simulink model "TosAeb"  (R2021a)
settings: Solver=FixedStepDiscrete  StartTime=0.0  StopTime=inf  FixedStep=0.01  RelTol=1e-3
undefined symbols (not defined in the file or in its folder): ACCModSts, AccSts1, AEB_ObjectMovingOrientation, AEB_ObjectMovingState, CN_BTCActive, CN_CurrentGearR, CN_CurrentGearR_DHT, CN_PTCActive, CN_VDCActive, CN_VSC_EspOff, CN_VSC_FCW_AEB_Error, CN_VSC_FCW_AEB_NoDispay, CN_VSC_FCW_AEB_PerfmDgdtn, CN_VSC_FCW_AEB_TempUnAvlbl, CN_VSC_VehStandstill, CNaccelAvoidMax, CNaccelAvoidMin, CNbrakePedalPress, CNcomfortStrLeftAccelMax, CNcomfortStrLeftAccelMin, CNcomfortStrRightAccelMax, CNcomfortStrRightAccelMin, CNDrvDoorOpen, CNDrvModeEco, CNDrvModeNorm, CNDrvModeOther, CNDrvModeSport, CNDrvSBRreminder, CNNull, CNobjInvalidID, CNPassDoorOpen, CNPassSBRreminder, CNPEBSAccelNegInf, CNPEBSAccelNull, CNPEBSTimeInf, CNPEBSTimeNull, CNSTOPMAX, CNSTOPNULL, CNsubjWidth, CNSwtOFF, CNSwtON, CNTTCMAX, CNTTCNULL, CNVehConfigAEBCycNone, CNVehConfigAEBNone, CNVehConfigAEBPedNone, CNVehConfigFCWNone, CNVehConfigJANone, CollisionSeverity, FALSE, FCWSnvty, JACycObjCalcInfoDefaultParams, JAPedObjCalcInfoDefaultParams, JAVehObjCalcInfoDefaultParams, latCycObjCalcInfoDefaultParams, latPedObjCalcInfoDefaultParams, longObjCalcInfoDefaultParams, ModSts, movingStatus, ObjectType ... (+1112)
libraries: simulink x3031, AEBSFunctionLib x50, Ta_Library x33, AEBSStateMachineLib x23, Mylibrary x12, simulink_extras x7, ObjSelect_lib x3, dspstat3 x2
(part 11 of 102)

== system TosAeb/FcwAebJa_Step_sys/ALG_AEBSystem/ALG_AEBOutput/AEBS_DVRInfoCalc/AEB_DVR_INFOMap ==
  AEB_LON_DVR_INFO : Inport
  AEB_LATPED_DVR_INFO : Inport  Port=2
  AEB_LATCYC_DVR_INFO : Inport  Port=3
  AEB_JAVEH_DVR_INFO : Inport  Port=4
  AEB_JAPED_DVR_INFO : Inport  Port=5
  Bus Creator : BusCreator  Inputs=62  OutDataTypeStr=Bus: AEB_DVR_INFO_BUS
      settings: InheritFromInputs=off
  Bus Selector : BusSelector  OutputSignals=AEBObjID,AEBObjVideoCfmd,AEBObjState,AEBObjDx,AEBObjDy,AEBObjVx,AEBObjVy,AEBObjAx,AEBObjAy,AEBObjExist,AEBObjObstcl,AEBObjCntAlive,AEBObjOverlap,AEBObjDrvby
  Bus Selector1 : BusSelector  OutputSignals=PedObjID,PedObjVideoCfmd,PedObjState,PedObjDx,PedObjDy,PedObjVx,PedObjVy,PedObjAx,PedObjAy,PedObjExist,PedObjObstcl,PedObjCntAlive
  Bus Selector2 : BusSelector  OutputSignals=CyclistObjID,CyclistObjVideoCfmd,CyclistObjState,CyclistObjDx,CyclistObjDy,CyclistObjVx,CyclistObjVy,CyclistObjAx,CyclistObjAy,CyclistObjExist,CyclistObjObstcl,CyclistObjCntAlive
  Bus Selector3 : BusSelector  OutputSignals=AEBJAObjID,AEBJAObjVideoCfmd,AEBJAObjState,AEBJAObjDx,AEBJAObjDy,AEBJAObjVx,AEBJAObjVy,AEBJAObjAx,AEBJAObjAy,AEBJAObjExist,AEBJAObjObstcl,AEBJAObjCntAlive
  Bus Selector4 : BusSelector  OutputSignals=JAPedObjID,JAPedObjVideoCfmd,JAPedObjState,JAPedObjDx,JAPedObjDy,JAPedObjVx,JAPedObjVy,JAPedObjAx,JAPedObjAy,JAPedObjExist,JAPedObjObstcl,JAPedObjCntAlive
  Data Type Conversion{1..2,6,8..14,16,20..31,34..45,48..56,59..63,65} (50 alike) : DataTypeConversion
  Data Type Conversion{7,15,19,32..33,46..47,57..58,64} (10 alike) : DataTypeConversion  OutDataTypeStr=uint16
  Data Type Conversion17 : DataTypeConversion  OutDataTypeStr=uint8
  Data Type Conversion18 : DataTypeConversion  OutDataTypeStr=uint8
  AEB_DVR_INFO : Outport  OutDataTypeStr=Bus: AEB_DVR_INFO_BUS
  wires:
  Bus Selector4/12 -> Data Type Conversion64/1  "<JAPedObjCntAlive>"
  Bus Selector4/11 -> Data Type Conversion65/1  "<JAPedObjObstcl>"
  Bus Selector4/10 -> Data Type Conversion60/1  "<JAPedObjExist>"
  Bus Selector4/9 -> Data Type Conversion63/1  "<JAPedObjAy>"
  Bus Selector4/8 -> Data Type Conversion62/1  "<JAPedObjAx>"
  Bus Selector4/7 -> Data Type Conversion61/1  "<JAPedObjVy>"
  Bus Selector4/6 -> Data Type Conversion52/1  "<JAPedObjVx>"
  Bus Selector4/5 -> Data Type Conversion55/1  "<JAPedObjDy>"
  Bus Selector4/4 -> Data Type Conversion54/1  "<JAPedObjDx>"
  Bus Selector4/3 -> Data Type Conversion53/1  "<JAPedObjState>"
  Bus Selector4/2 -> Data Type Conversion51/1  "<JAPedObjVideoCfmd>"
  Bus Selector4/1 -> Data Type Conversion58/1  "<JAPedObjID>"
  AEB_JAPED_DVR_INFO/1 -> Bus Selector4/1
  Bus Selector3/12 -> Data Type Conversion57/1  "<AEBJAObjCntAlive>"
  Bus Selector3/11 -> Data Type Conversion56/1  "<AEBJAObjObstcl>"
  Bus Selector3/10 -> Data Type Conversion59/1  "<AEBJAObjExist>"
  Bus Selector3/9 -> Data Type Conversion50/1  "<AEBJAObjAy>"
  Bus Selector3/8 -> Data Type Conversion49/1  "<AEBJAObjAx>"
  Bus Selector3/7 -> Data Type Conversion48/1  "<AEBJAObjVy>"
  Bus Selector3/6 -> Data Type Conversion41/1  "<AEBJAObjVx>"
  Bus Selector3/5 -> Data Type Conversion44/1  "<AEBJAObjDy>"
  Bus Selector3/4 -> Data Type Conversion43/1  "<AEBJAObjDx>"
  Bus Selector3/3 -> Data Type Conversion42/1  "<AEBJAObjState>"
  Bus Selector3/2 -> Data Type Conversion40/1  "<AEBJAObjVideoCfmd>"
  Bus Selector3/1 -> Data Type Conversion47/1  "<AEBJAObjID>"
  AEB_JAVEH_DVR_INFO/1 -> Bus Selector3/1
  Bus Selector2/12 -> Data Type Conversion46/1  "<CyclistObjCntAlive>"
  Bus Selector2/11 -> Data Type Conversion45/1  "<CyclistObjObstcl>"
  Bus Selector2/10 -> Data Type Conversion6/1  "<CyclistObjExist>"
  Bus Selector2/9 -> Data Type Conversion39/1  "<CyclistObjAy>"
  Bus Selector2/8 -> Data Type Conversion38/1  "<CyclistObjAx>"
  Bus Selector2/7 -> Data Type Conversion37/1  "<CyclistObjVy>"
  Bus Selector2/6 -> Data Type Conversion27/1  "<CyclistObjVx>"
  Bus Selector2/5 -> Data Type Conversion30/1  "<CyclistObjDy>"
  Bus Selector2/4 -> Data Type Conversion29/1  "<CyclistObjDx>"
  Bus Selector2/3 -> Data Type Conversion28/1  "<CyclistObjState>"
  Bus Selector2/2 -> Data Type Conversion26/1  "<CyclistObjVideoCfmd>"
  Bus Selector2/1 -> Data Type Conversion33/1  "<CyclistObjID>"
  AEB_LATCYC_DVR_INFO/1 -> Bus Selector2/1
  Bus Selector1/12 -> Data Type Conversion32/1  "<PedObjCntAlive>"
  Bus Selector1/11 -> Data Type Conversion31/1  "<PedObjObstcl>"
  Bus Selector1/10 -> Data Type Conversion2/1  "<PedObjExist>"
  Bus Selector1/9 -> Data Type Conversion25/1  "<PedObjAy>"
  Bus Selector1/8 -> Data Type Conversion24/1  "<PedObjAx>"
  Bus Selector1/7 -> Data Type Conversion23/1  "<PedObjVy>"
  Bus Selector1/6 -> Data Type Conversion1/1  "<PedObjVx>"
  Bus Selector1/1 -> Data Type Conversion19/1  "<PedObjID>"
  Bus Selector1/5 -> Data Type Conversion36/1  "<PedObjDy>"
  Bus Selector1/2 -> Data Type Conversion16/1  "<PedObjVideoCfmd>"
  Bus Selector1/4 -> Data Type Conversion35/1  "<PedObjDx>"
  Bus Selector1/3 -> Data Type Conversion34/1  "<PedObjState>"
  AEB_LATPED_DVR_INFO/1 -> Bus Selector1/1
  Bus Selector/14 -> Data Type Conversion18/1  "<AEBObjDrvby>"
  Bus Selector/13 -> Data Type Conversion17/1  "<AEBObjOverlap>"
  Bus Selector/12 -> Data Type Conversion15/1  "<AEBObjCntAlive>"
  Bus Selector/11 -> Data Type Conversion22/1  "<AEBObjObstcl>"
  Bus Selector/10 -> Data Type Conversion21/1  "<AEBObjExist>"
  Bus Selector/9 -> Data Type Conversion20/1  "<AEBObjAy>"
  Bus Selector/8 -> Data Type Conversion11/1  "<AEBObjAx>"
  Bus Selector/7 -> Data Type Conversion14/1  "<AEBObjVy>"
  Bus Selector/6 -> Data Type Conversion13/1  "<AEBObjVx>"
  Bus Selector/5 -> Data Type Conversion12/1  "<AEBObjDy>"
  Bus Selector/4 -> Data Type Conversion10/1  "<AEBObjDx>"
  Bus Selector/3 -> Data Type Conversion9/1  "<AEBObjState>"
  Bus Selector/2 -> Data Type Conversion8/1  "<AEBObjVideoCfmd>"
  Bus Selector/1 -> Data Type Conversion7/1  "<AEBObjID>"
  AEB_LON_DVR_INFO/1 -> Bus Selector/1
  Data Type Conversion24/1 -> Bus Creator/22  "PedObjAx"
  Data Type Conversion16/1 -> Bus Creator/16  "PedObjVideoCfmd"
  Data Type Conversion20/1 -> Bus Creator/9  "AEBObjAy"
  Data Type Conversion47/1 -> Bus Creator/39  "AEBJAObjID"
  Data Type Conversion38/1 -> Bus Creator/34  "CyclistObjAx"
  Data Type Conversion11/1 -> Bus Creator/8  "AEBObjAx"
  Data Type Conversion17/1 -> Bus Creator/13  "AEBObjOverlap"
  Data Type Conversion55/1 -> Bus Creator/55  "JAPedObjDy"
  Data Type Conversion22/1 -> Bus Creator/11  "AEBObjObstcl"
  Data Type Conversion19/1 -> Bus Creator/15  "PedObjID"
  Data Type Conversion9/1 -> Bus Creator/3  "AEBObjState"
  Data Type Conversion53/1 -> Bus Creator/53  "JAPedObjState"
  Data Type Conversion60/1 -> Bus Creator/60  "JAPedObjExist"
  Data Type Conversion33/1 -> Bus Creator/27  "CyclistObjID"
  Data Type Conversion56/1 -> Bus Creator/49  "AEBJAObjObstcl"
  Data Type Conversion36/1 -> Bus Creator/19  "PedObjDy"
  Data Type Conversion57/1 -> Bus Creator/50  "AEBJAObjCntAlive"
  Data Type Conversion6/1 -> Bus Creator/36  "CyclistObjExist"
  Data Type Conversion10/1 -> Bus Creator/4  "AEBObjDx"
  Data Type Conversion52/1 -> Bus Creator/56  "JAPedObjVx"
  Data Type Conversion8/1 -> Bus Creator/2  "AEBObjVideoCfmd"
  Data Type Conversion15/1 -> Bus Creator/12  "AEBObjCntAlive"
  Data Type Conversion18/1 -> Bus Creator/14  "AEBObjDrvby"
  Data Type Conversion41/1 -> Bus Creator/44  "AEBJAObjVx"
  Data Type Conversion21/1 -> Bus Creator/10  "AEBObjExist"
  Bus Creator/1 -> AEB_DVR_INFO/1
  Data Type Conversion14/1 -> Bus Creator/7  "AEBObjVy"
  Data Type Conversion34/1 -> Bus Creator/17  "PedObjState"
  Data Type Conversion23/1 -> Bus Creator/21  "PedObjVy"
  Data Type Conversion42/1 -> Bus Creator/41  "AEBJAObjState"
  Data Type Conversion2/1 -> Bus Creator/24  "PedObjExist"
  Data Type Conversion35/1 -> Bus Creator/18  "PedObjDx"
  Data Type Conversion31/1 -> Bus Creator/25  "PedObjObstcl"
  Data Type Conversion28/1 -> Bus Creator/29  "CyclistObjState"
  Data Type Conversion7/1 -> Bus Creator/1  "AEBObjID"
  Data Type Conversion49/1 -> Bus Creator/46  "AEBJAObjAx"
  Data Type Conversion61/1 -> Bus Creator/57  "JAPedObjVy"
  Data Type Conversion39/1 -> Bus Creator/35  "CyclistObjAy"
  Data Type Conversion59/1 -> Bus Creator/48  "AEBJAObjExist"
  Data Type Conversion62/1 -> Bus Creator/58  "JAPedObjAx"
  Data Type Conversion43/1 -> Bus Creator/42  "AEBJAObjDx"
  Data Type Conversion46/1 -> Bus Creator/38  "CyclistObjCntAlive"
  Data Type Conversion44/1 -> Bus Creator/43  "AEBJAObjDy"
  Data Type Conversion30/1 -> Bus Creator/31  "CyclistObjDy"
  Data Type Conversion63/1 -> Bus Creator/59  "JAPedObjAy"
  Data Type Conversion50/1 -> Bus Creator/47  "AEBJAObjAy"
  Data Type Conversion27/1 -> Bus Creator/32  "CyclistObjVx"
  Data Type Conversion45/1 -> Bus Creator/37  "CyclistObjObstcl"
  Data Type Conversion26/1 -> Bus Creator/28  "CyclistObjVideoCfmd"
  Data Type Conversion65/1 -> Bus Creator/61  "JAPedObjObstcl"
  Data Type Conversion13/1 -> Bus Creator/6  "AEBObjVx"
  Data Type Conversion29/1 -> Bus Creator/30  "CyclistObjDx"
  Data Type Conversion32/1 -> Bus Creator/26  "PedObjCntAlive"
  Data Type Conversion40/1 -> Bus Creator/40  "AEBJAObjVideoCfmd"
  Data Type Conversion64/1 -> Bus Creator/62  "JAPedObjCntAlive"
  Data Type Conversion12/1 -> Bus Creator/5  "AEBObjDy"
  Data Type Conversion58/1 -> Bus Creator/51  "JAPedObjID"
  Data Type Conversion25/1 -> Bus Creator/23  "PedObjAy"
  Data Type Conversion48/1 -> Bus Creator/45  "AEBJAObjVy"
  Data Type Conversion1/1 -> Bus Creator/20  "PedObjVx"
  Data Type Conversion54/1 -> Bus Creator/54  "JAPedObjDx"
  Data Type Conversion51/1 -> Bus Creator/52  "JAPedObjVideoCfmd"
  Data Type Conversion37/1 -> Bus Creator/33  "CyclistObjVy"

== system TosAeb/FcwAebJa_Step_sys/ALG_AEBSystem/ALG_AEBOutput/AEBS_DVRInfoCalc/AEB_JAPED_DVR_INFO ==
  VehConfigAEBJA : Inport
  JAPedTgtCalcInfo : Inport  Port=2
  Compare To Constant : simulink/Logic and Bit Operations/Compare To Constant  relop===  const=FALSE  OutDataTypeStr=boolean
  If : If  IfExpression=u1
  If Action Subsystem : Subsystem  TreatAsAtomicUnit=on
  Merge : Merge
  Subsystem : Subsystem
  AEB_JAPED_DVR_INFO : Outport
  wires:
  Merge/1 -> AEB_JAPED_DVR_INFO/1
  Subsystem/1(AEB_JAPED_DVR_INFO) -> Merge/2
  If Action Subsystem/1(AEB_JAPED_DVR_INFO) -> Merge/1
  If/2 -> Subsystem/Action
  If/1 -> If Action Subsystem/Action
  Compare To Constant/1 -> If/1
  VehConfigAEBJA/1 -> Compare To Constant/1
  JAPedTgtCalcInfo/1 -> Subsystem/1(JAPedTgtCalcInfo)

== system TosAeb/FcwAebJa_Step_sys/ALG_AEBSystem/ALG_AEBOutput/AEBS_DVRInfoCalc/AEB_JAVEH_DVR_INFO ==
  JAVehTgtCalcInfo : Inport
  VehConfigAEBJA : Inport  Port=2
  Compare To Constant : simulink/Logic and Bit Operations/Compare To Constant  relop===  const=FALSE  OutDataTypeStr=boolean
  If : If  IfExpression=u1
  If Action Subsystem : Subsystem  TreatAsAtomicUnit=on
  Merge : Merge
  Subsystem : Subsystem
  AEB_JAVEH_DVR_INFO : Outport
  wires:
  Merge/1 -> AEB_JAVEH_DVR_INFO/1
  Subsystem/1(AEB_JAVEH_DVR_INFO) -> Merge/2
  If Action Subsystem/1(AEB_JAVEH_DVR_INFO) -> Merge/1
  If/2 -> Subsystem/Action
  If/1 -> If Action Subsystem/Action
  JAVehTgtCalcInfo/1 -> Subsystem/1(JAVehTgtCalcInfo)
  Compare To Constant/1 -> If/1
  VehConfigAEBJA/1 -> Compare To Constant/1

== system TosAeb/FcwAebJa_Step_sys/ALG_AEBSystem/ALG_AEBOutput/AEBS_DVRInfoCalc/AEB_LATCYC_DVR_INFO ==
  VehicleConfigAEBCyc : Inport
  latCycTgtCalcInfo : Inport  Port=2
  Compare To Constant : simulink/Logic and Bit Operations/Compare To Constant  relop===  const=FALSE  OutDataTypeStr=boolean
  If : If  IfExpression=u1
  If Action Subsystem : Subsystem  TreatAsAtomicUnit=on
  Merge : Merge
  Subsystem : Subsystem
  AEB_LATCYC_DVR_INFO : Outport
  wires:
  Merge/1 -> AEB_LATCYC_DVR_INFO/1
  Subsystem/1(AEB_LATCYC_DVR_INFO) -> Merge/2
  If Action Subsystem/1(AEB_LATCYC_DVR_INFO) -> Merge/1
  If/2 -> Subsystem/Action
  If/1 -> If Action Subsystem/Action
  Compare To Constant/1 -> If/1
  VehicleConfigAEBCyc/1 -> Compare To Constant/1
  latCycTgtCalcInfo/1 -> Subsystem/1(latCycTgtCalcInfo)

== system TosAeb/FcwAebJa_Step_sys/ALG_AEBSystem/ALG_AEBOutput/AEBS_DVRInfoCalc/AEB_LATPED_DVR_INFO ==
  VehicleConfigAEBPed : Inport
  latPedTgtCalcInfo : Inport  Port=2
  Compare To Constant : simulink/Logic and Bit Operations/Compare To Constant  relop===  const=FALSE  OutDataTypeStr=boolean
  If : If  IfExpression=u1
  If Action Subsystem : Subsystem  TreatAsAtomicUnit=on
  Merge : Merge
  Subsystem : Subsystem
  AEB_LATPED_DVR_INFO : Outport
  wires:
  Merge/1 -> AEB_LATPED_DVR_INFO/1
  Subsystem/1(AEB_LATPED_DVR_INFO) -> Merge/2
  If Action Subsystem/1(AEB_LATPED_DVR_INFO) -> Merge/1
  If/2 -> Subsystem/Action
  If/1 -> If Action Subsystem/Action
  VehicleConfigAEBPed/1 -> Compare To Constant/1
  Compare To Constant/1 -> If/1
  latPedTgtCalcInfo/1 -> Subsystem/1(latPedTgtCalcInfo)

== system TosAeb/FcwAebJa_Step_sys/ALG_AEBSystem/ALG_AEBOutput/AEBS_DVRInfoCalc/AEB_LON_DVR_INFO ==
  VehicleConfigFCW : Inport
  VehicleConfigAEB : Inport  Port=2
  longObjCalcInfo : Inport  Port=3
  Compare To Constant : simulink/Logic and Bit Operations/Compare To Constant  relop===  const=FALSE  OutDataTypeStr=boolean
  If : If  IfExpression=u1
  If Action Subsystem : Subsystem  TreatAsAtomicUnit=on
  If Action Subsystem1 : Subsystem  TreatAsAtomicUnit=on
  Logical Operator : Logic  Operator=OR  AllPortsSameDT=off  OutDataTypeStr=boolean
  Merge : Merge
  AEB_LON_DVR_INFO : Outport
  wires:
  Compare To Constant/1 -> If/1
  VehicleConfigAEB/1 -> Logical Operator/2
  Logical Operator/1 -> Compare To Constant/1
  VehicleConfigFCW/1 -> Logical Operator/1
  If Action Subsystem/1(AEB_LON_DVR_INFO) -> Merge/2
  longObjCalcInfo/1 -> If Action Subsystem/1(longObjCalcInfo)
  If Action Subsystem1/1(AEB_LON_DVR_INFO) -> Merge/1
  If/2 -> If Action Subsystem/Action
  If/1 -> If Action Subsystem1/Action
  Merge/1 -> AEB_LON_DVR_INFO/1

== system TosAeb/FcwAebJa_Step_sys/ALG_AEBSystem/ALG_AEBOutput/AEBS_IPHUTInfoCalc/AEBS_IPHUTInfoMap ==
  note: AEB_LePedDetn
      |
      | AEB_LePedTyp
      |
      | AEB_LePedHeading
      |
      | AEB_LePedDx
      |
      | AEB_LePedDy
      |
      | AEB_LePed_collisionprob
      |
      | AEB_RiPedDetn
      |
      | AEB_RiPedTyp
      |
      | AEB_RiPedHeading
      |
      | AEB_RiPedDx
      |
      | AEB_RiPedDy
      |
      | AEB_RiPed_collisionprob
      |
      | AEB_LeCyclistDetn
      |
      | AEB_LeCyclistTyp
      |
      | AEB_LeCyclistHeading
      |
      | AEB_LeCyclistDx
      |
      | AEB_LeCyclistDy
      |
      | AEB_LeCyclist_collisionprob
      |
      | AEB_RiCyclistDetn
      |
      | AEB_RiCyclistTyp
      |
      | AEB_RiCyclistHeading
      |
      | AEB_RiCyclistDx
      |
      | AEB_RiCyclistDy
      |
      | AEB_RiCyclist_collisionprob
      |
      | FCWAEB_longTTC
      |
      | FCWAEB_crossingTTC
      |
      | FCWAEB_JATTC
  note: 0x0:No Warning
      | 0x1:Warning
  note: 0x0:No Warning
      | 0x1:LattenWarning
  AEB_VehResp : Inport
  AEB_PedResp : Inport  Port=2
  FCWSnvty : Inport  Port=3
  AEB_JAResp : Inport  Port=4
  FCW_AEB_VehFctSts : Inport  Port=5
  FCW_AEB_PedFctSts : Inport  Port=6
  FCW_AEB_JAFctSts : Inport  Port=7
  AEB_VehTrig : Inport  Port=8
  AEB_PedTrig : Inport  Port=9
  AEB_JABrkTrig : Inport  Port=10
  AEB_ObjSty : Inport  Port=11
  FCW_Warn : Inport  Port=12
  Latten_Warn : Inport  Port=13
  AR_HUD_Info : Inport  Port=14
  Bus Creator : BusCreator  Inputs=40  OutDataTypeStr=Bus: AEB_IPHUT_INFO_BUS  NonVirtualBus=on
      settings: InheritFromInputs=off
  Bus Selector : BusSelector  OutputSignals=AEB_LePedDetn,AEB_LePedTyp,AEB_LePedHeading,AEB_LePedDx,AEB_LePedDy,AEB_LePed_collisionprob,AEB_RiPedDetn,AEB_RiPedTyp,AEB_RiPedHeading,AEB_RiPedDx,AEB_RiPedDy,AEB_RiPed_collisionprob,AEB_LeCyclistDetn,AEB_LeCyclistTyp,AEB_LeCyclistHeading,AEB_LeCyclistDx,AEB_LeCyclistDy,AEB_LeCyclist_collisionprob,AEB_RiCyclistDetn,AEB_RiCyclistTyp,AEB_RiCyclistHeading,AEB_RiCyclistDx,AEB_RiCyclistDy,AEB_RiCyclist_collisionprob,FCWAEB_longTTC,FCWAEB_crossingTTC,FCWAEB_JATTC
  Data Type Conversion{,1..5,34} (7 alike) : DataTypeConversion  OutDataTypeStr=uint8  SaturateOnIntegerOverflow=on
  Data Type Conversion{6..33} (28 alike) : DataTypeConversion
  Signal Conversion{,1..39} (40 alike) : SignalConversion  OverrideOpt=off
  AEB_IPHUT_INFO : Outport  OutDataTypeStr=Bus: AEB_IPHUT_INFO_BUS
  wires:
  Bus Selector/27 -> Data Type Conversion32/1  "<FCWAEB_JATTC>"
  Bus Selector/26 -> Data Type Conversion31/1  "<FCWAEB_crossingTTC>"
  Bus Selector/25 -> Data Type Conversion26/1  "<FCWAEB_longTTC>"
  Bus Selector/24 -> Data Type Conversion30/1  "<AEB_RiCyclist_collisionprob>"
  Bus Selector/23 -> Data Type Conversion29/1  "<AEB_RiCyclistDy>"
  Bus Selector/22 -> Data Type Conversion28/1  "<AEB_RiCyclistDx>"
  Bus Selector/21 -> Data Type Conversion27/1  "<AEB_RiCyclistHeading>"
  Bus Selector/20 -> Data Type Conversion21/1  "<AEB_RiCyclistTyp>"
  Bus Selector/19 -> Data Type Conversion25/1  "<AEB_RiCyclistDetn>"
  Bus Selector/18 -> Data Type Conversion24/1  "<AEB_LeCyclist_collisionprob>"
  Bus Selector/17 -> Data Type Conversion23/1  "<AEB_LeCyclistDy>"
  Bus Selector/16 -> Data Type Conversion22/1  "<AEB_LeCyclistDx>"
  Bus Selector/15 -> Data Type Conversion16/1  "<AEB_LeCyclistHeading>"
  Bus Selector/14 -> Data Type Conversion20/1  "<AEB_LeCyclistTyp>"
  Bus Selector/13 -> Data Type Conversion19/1  "<AEB_LeCyclistDetn>"
  Bus Selector/12 -> Data Type Conversion18/1  "<AEB_RiPed_collisionprob>"
  Bus Selector/11 -> Data Type Conversion17/1  "<AEB_RiPedDy>"
  Bus Selector/10 -> Data Type Conversion11/1  "<AEB_RiPedDx>"
  Bus Selector/9 -> Data Type Conversion15/1  "<AEB_RiPedHeading>"
  Bus Selector/8 -> Data Type Conversion14/1  "<AEB_RiPedTyp>"
  Bus Selector/7 -> Data Type Conversion13/1  "<AEB_RiPedDetn>"
  Bus Selector/6 -> Data Type Conversion12/1  "<AEB_LePed_collisionprob>"
  Bus Selector/5 -> Data Type Conversion10/1  "<AEB_LePedDy>"
  Bus Selector/4 -> Data Type Conversion9/1  "<AEB_LePedDx>"
  Bus Selector/3 -> Data Type Conversion8/1  "<AEB_LePedHeading>"
  Bus Selector/2 -> Data Type Conversion7/1  "<AEB_LePedTyp>"
  Bus Selector/1 -> Data Type Conversion6/1  "<AEB_LePedDetn>"
  AR_HUD_Info/1 -> Bus Selector/1
  Data Type Conversion33/1 -> Signal Conversion12/1
  Data Type Conversion32/1 -> Signal Conversion39/1
  Data Type Conversion31/1 -> Signal Conversion38/1
  Data Type Conversion30/1 -> Signal Conversion36/1
  Data Type Conversion28/1 -> Signal Conversion34/1
  Data Type Conversion29/1 -> Signal Conversion35/1
  Data Type Conversion26/1 -> Signal Conversion37/1
  Data Type Conversion27/1 -> Signal Conversion33/1
  Data Type Conversion23/1 -> Signal Conversion29/1
  Data Type Conversion22/1 -> Signal Conversion28/1
  Data Type Conversion24/1 -> Signal Conversion30/1
  Data Type Conversion21/1 -> Signal Conversion32/1
  Data Type Conversion25/1 -> Signal Conversion31/1
  Data Type Conversion18/1 -> Signal Conversion24/1
  Data Type Conversion19/1 -> Signal Conversion25/1
  Data Type Conversion17/1 -> Signal Conversion23/1
  Data Type Conversion16/1 -> Signal Conversion27/1
  Data Type Conversion20/1 -> Signal Conversion26/1
  Data Type Conversion11/1 -> Signal Conversion22/1
  Data Type Conversion12/1 -> Signal Conversion18/1
  Data Type Conversion14/1 -> Signal Conversion20/1
  Data Type Conversion13/1 -> Signal Conversion19/1
  Data Type Conversion15/1 -> Signal Conversion21/1
  Data Type Conversion10/1 -> Signal Conversion17/1
  Data Type Conversion9/1 -> Signal Conversion16/1
  Data Type Conversion8/1 -> Signal Conversion15/1
  Data Type Conversion7/1 -> Signal Conversion14/1
  Data Type Conversion6/1 -> Signal Conversion13/1
  Signal Conversion39/1 -> Bus Creator/40  "FCWAEB_JATTC"
  Signal Conversion35/1 -> Bus Creator/36  "AEB_RiCyclistDy"
  Signal Conversion36/1 -> Bus Creator/37  "AEB_RiCyclist_collisionprob"
  Signal Conversion37/1 -> Bus Creator/38  "FCWAEB_longTTC"
  Signal Conversion38/1 -> Bus Creator/39  "FCWAEB_crossingTTC"
  Signal Conversion34/1 -> Bus Creator/35  "AEB_RiCyclistDx"
  Signal Conversion33/1 -> Bus Creator/34  "AEB_RiCyclistHeading"
  Signal Conversion32/1 -> Bus Creator/33  "AEB_RiCyclistTyp"
  Signal Conversion31/1 -> Bus Creator/32  "AEB_RiCyclistDetn"
  Signal Conversion30/1 -> Bus Creator/31  "AEB_LeCyclist_collisionprob"
  Signal Conversion29/1 -> Bus Creator/30  "AEB_LeCyclistDy"
  Signal Conversion28/1 -> Bus Creator/29  "AEB_LeCyclistDx"
  Signal Conversion27/1 -> Bus Creator/28  "AEB_LeCyclistHeading"
  Signal Conversion26/1 -> Bus Creator/27  "AEB_LeCyclistTyp"
  Signal Conversion25/1 -> Bus Creator/26  "AEB_LeCyclistDetn"
  Signal Conversion24/1 -> Bus Creator/25  "AEB_RiPed_collisionprob"
  Signal Conversion23/1 -> Bus Creator/24  "AEB_RiPedDy"
  Signal Conversion22/1 -> Bus Creator/23  "AEB_RiPedDx"
  Signal Conversion21/1 -> Bus Creator/22  "AEB_RiPedHeading"
  Signal Conversion20/1 -> Bus Creator/21  "AEB_RiPedTyp"
  Signal Conversion19/1 -> Bus Creator/20  "AEB_RiPedDetn"
  Signal Conversion18/1 -> Bus Creator/19  "AEB_LePed_collisionprob"
  Signal Conversion17/1 -> Bus Creator/18  "AEB_LePedDy"
  Signal Conversion16/1 -> Bus Creator/17  "AEB_LePedDx"
  Signal Conversion15/1 -> Bus Creator/16  "AEB_LePedHeading"
  Signal Conversion14/1 -> Bus Creator/15  "AEB_LePedTyp"
  Signal Conversion13/1 -> Bus Creator/14  "AEB_LePedDetn"
  Data Type Conversion5/1 -> Signal Conversion6/1
  Data Type Conversion4/1 -> Signal Conversion10/1
  Data Type Conversion2/1 -> Signal Conversion9/1
  Data Type Conversion/1 -> Signal Conversion8/1
  Data Type Conversion3/1 -> Signal Conversion7/1
  Data Type Conversion1/1 -> Signal Conversion2/1
  Data Type Conversion34/1 -> Signal Conversion11/1
  AEB_VehTrig/1 -> Signal Conversion4/1
  FCW_Warn/1 -> Data Type Conversion2/1
  AEB_PedTrig/1 -> Signal Conversion5/1
  FCW_AEB_VehFctSts/1 -> Data Type Conversion3/1
  AEB_VehResp/1 -> Signal Conversion/1
  AEB_JAResp/1 -> Signal Conversion3/1
  AEB_PedResp/1 -> Signal Conversion1/1
  FCWSnvty/1 -> Data Type Conversion1/1
  FCW_AEB_JAFctSts/1 -> Data Type Conversion4/1
  FCW_AEB_PedFctSts/1 -> Data Type Conversion/1
  AEB_ObjSty/1 -> Data Type Conversion5/1
  Signal Conversion12/1 -> Bus Creator/10  "AEB_JABrkTrig"
  AEB_JABrkTrig/1 -> Data Type Conversion33/1
  Signal Conversion11/1 -> Bus Creator/12  "Latten_Warn"
  Signal Conversion10/1 -> Bus Creator/7  "AEB_JAFuncSts"
  Signal Conversion1/1 -> Bus Creator/2  "AEB_PedResp"
  Signal Conversion5/1 -> Bus Creator/9  "AEB_PedTrig"
  Bus Creator/1 -> AEB_IPHUT_INFO/1
  Signal Conversion9/1 -> Bus Creator/11  "FCW_Warn"
  Signal Conversion8/1 -> Bus Creator/6  "FCW_AEB_PedFctSts"
  Signal Conversion2/1 -> Bus Creator/4  "FCW_Snvty"
  Signal Conversion7/1 -> Bus Creator/5  "FCW_AEB_VehFctSts"
  Signal Conversion3/1 -> Bus Creator/3  "AEB_JA_Resp"
  Signal Conversion6/1 -> Bus Creator/13  "AEB_ObjSty"
  Signal Conversion/1 -> Bus Creator/1  "AEB_VehResp"
  Signal Conversion4/1 -> Bus Creator/8  "AEB_VehTrig"
  Latten_Warn/1 -> Data Type Conversion34/1

== system TosAeb/FcwAebJa_Step_sys/ALG_AEBSystem/ALG_AEBOutput/AEBS_IPHUTInfoCalc/AEB_ObjStyMap ==
  AEBTrigger : Inport
  AEBpartFuncImpArb : Inport  Port=2
  AEBfullFuncImpArb : Inport  Port=3
  EBAFuncImpArb : Inport  Port=4
  cycAEBpartFuncImpArb : Inport  Port=5
  cycAEBfullFuncImpArb : Inport  Port=6
  pedAEBfullFuncImpArb : Inport  Port=7
  JA_AEBfullFuncImpArb : Inport  Port=8
  JA_pedAEBfullFuncImpArb : Inport  Port=9
  longObjectType : Inport  Port=10
  latPedObjectType : Inport  Port=11
  latCycObjectType : Inport  Port=12
  JaPedObjectType : Inport  Port=13
  JaVehObjectType : Inport  Port=14
  AEB_ObjStyFlow : Stateflow chart  TreatAsAtomicUnit=on
  AEB_ObjSty : Outport
  wires:
  JaVehObjectType/1 -> AEB_ObjStyFlow/14(JaVehObjectType)
  JaPedObjectType/1 -> AEB_ObjStyFlow/13(JaPedObjectType)
  latCycObjectType/1 -> AEB_ObjStyFlow/12(latCycObjectType)
  latPedObjectType/1 -> AEB_ObjStyFlow/11(latPedObjectType)
  longObjectType/1 -> AEB_ObjStyFlow/10(longObjectType)
  AEB_ObjStyFlow/1(Inter_AEB_ObjSty) -> AEB_ObjSty/1
  cycAEBfullFuncImpArb/1 -> AEB_ObjStyFlow/6(cycAEBpartFuncImpArb)
  cycAEBpartFuncImpArb/1 -> AEB_ObjStyFlow/5(pedAEBfullFuncImpArb)
  EBAFuncImpArb/1 -> AEB_ObjStyFlow/4(AEBfullFuncImpArb)
  AEBfullFuncImpArb/1 -> AEB_ObjStyFlow/3(EBAfullFuncImpArb)
  AEBTrigger/1 -> AEB_ObjStyFlow/1(AEBTrigger)
  AEBpartFuncImpArb/1 -> AEB_ObjStyFlow/2(AEBPartFuncImpArb)
  JA_AEBfullFuncImpArb/1 -> AEB_ObjStyFlow/8(JA_AEBfullFuncImpArb)
  JA_pedAEBfullFuncImpArb/1 -> AEB_ObjStyFlow/9(JA_pedAEBfullFuncImpArb)
  pedAEBfullFuncImpArb/1 -> AEB_ObjStyFlow/7(cycAEBfullFuncImpArb)

== system TosAeb/FcwAebJa_Step_sys/ALG_AEBSystem/ALG_AEBOutput/AEBS_IPHUTInfoCalc/AEB_ObjStyMap1 ==
  AEBTrigger : Inport
  AEBpartFuncImpArb : Inport  Port=2
  AEBfullFuncImpArb : Inport  Port=3
  EBAFuncImpArb : Inport  Port=4
  cycAEBpartFuncImpArb : Inport  Port=5
  cycAEBfullFuncImpArb : Inport  Port=6
  pedAEBfullFuncImpArb : Inport  Port=7
  JA_AEBfullFuncImpArb : Inport  Port=8
  JA_pedAEBfullFuncImpArb : Inport  Port=9
  FCTBFuncImpArb : Inport  Port=10
  AEB_ObjStyFlow : Stateflow chart  TreatAsAtomicUnit=on
  AEB_ObjSty : Outport
  wires:
  FCTBFuncImpArb/1 -> AEB_ObjStyFlow/10(FCTBFuncImpArb)
  pedAEBfullFuncImpArb/1 -> AEB_ObjStyFlow/7(cycAEBfullFuncImpArb)
  JA_pedAEBfullFuncImpArb/1 -> AEB_ObjStyFlow/9(JA_pedAEBfullFuncImpArb)
  JA_AEBfullFuncImpArb/1 -> AEB_ObjStyFlow/8(JA_AEBfullFuncImpArb)
  AEBpartFuncImpArb/1 -> AEB_ObjStyFlow/2(AEBPartFuncImpArb)
  AEBTrigger/1 -> AEB_ObjStyFlow/1(AEBTrigger)
  AEBfullFuncImpArb/1 -> AEB_ObjStyFlow/3(EBAfullFuncImpArb)
  EBAFuncImpArb/1 -> AEB_ObjStyFlow/4(AEBfullFuncImpArb)
  cycAEBpartFuncImpArb/1 -> AEB_ObjStyFlow/5(pedAEBfullFuncImpArb)
  cycAEBfullFuncImpArb/1 -> AEB_ObjStyFlow/6(cycAEBpartFuncImpArb)
  AEB_ObjStyFlow/1(AEB_ObjSty) -> AEB_ObjSty/1

== system TosAeb/FcwAebJa_Step_sys/ALG_AEBSystem/ALG_AEBOutput/AEBS_IPHUTInfoCalc/AEB_TrigMap ==
  note: 0x0:Not tirggered
      | 0x1:AEB_JA Function Triggered for vehicle
      | 0x2:AEB_JA Function Triggered for pedestrian
      | 0x3:reverse
  note: AEB_VehTrigMap
  note: AEB_PedTrigMap
  note: AEB_JABrkTrigMap
  AEBTrigger : Inport
  AEBLonObjectType : Inport  Port=2
  EBAfullFuncImpArb : Inport  Port=3
  AEBfullFuncImpArb : Inport  Port=4
  AEBPartFuncImpArb : Inport  Port=5
  pedAEBfullFuncImpArb : Inport  Port=6
  cycAEBfullFuncImpArb : Inport  Port=7
  cycAEBpartFuncImpArb : Inport  Port=8
  JA_pedAEBfullFuncImpArb : Inport  Port=9
  JA_AEBfullFuncImpArb : Inport  Port=10
  Compare To Constant{,1..2,4..6,9..10} (8 alike) : simulink/Logic and Bit Operations/Compare To Constant  relop===  const=ModSts.ActiveState  OutDataTypeStr=boolean
  Compare To Constant{7..8,11,14} (4 alike) : simulink/Logic and Bit Operations/Compare To Constant  relop===  const=TRUE  OutDataTypeStr=boolean
  Compare To Constant12 : simulink/Logic and Bit Operations/Compare To Constant  relop===  const=ObjectType.BICYCLE  OutDataTypeStr=boolean
  Compare To Constant13 : simulink/Logic and Bit Operations/Compare To Constant  relop===  const=FALSE  OutDataTypeStr=boolean
  Compare To Constant3 : simulink/Logic and Bit Operations/Compare To Constant  relop===  const=ObjectType.PEDESTRIAN  OutDataTypeStr=boolean
  Constant : Constant  Value=0  OutDataTypeStr=uint8
  Constant1 : Constant  OutDataTypeStr=uint8
  Constant2 : Constant  Value=2  OutDataTypeStr=uint8
  Constant3 : Constant  Value=0  OutDataTypeStr=uint8
  Logical Operator : Logic  Operator=OR  Inputs=3  AllPortsSameDT=off  OutDataTypeStr=boolean
  Logical Operator{1,3,5..6} (4 alike) : Logic  AllPortsSameDT=off  OutDataTypeStr=boolean
  Logical Operator2 : Logic  Operator=OR  Inputs=3  AllPortsSameDT=off  OutDataTypeStr=boolean
  Logical Operator4 : Logic  Operator=OR  AllPortsSameDT=off  OutDataTypeStr=boolean
  Logical Operator7 : Logic  Operator=OR  AllPortsSameDT=off  OutDataTypeStr=boolean
  Switch : Switch  Criteria=u2 ~= 0
  Switch1 : Switch  Criteria=u2 ~= 0
  Switch2 : Switch  Criteria=u2 ~= 0
  AEB_VehTrig : Outport
  AEB_PedTrig : Outport  Port=2
  AEB_JABrkTrig : Outport  Port=3
  wires:
  [AEB_VehTrig] -> Logical Operator6/2
  Compare To Constant13/1 -> Logical Operator5/2
  Logical Operator1/1 -> Logical Operator5/1, [AEB_VehTrig]
  Compare To Constant14/1 -> Logical Operator6/1
  Logical Operator6/1 -> Logical Operator7/1
  Logical Operator7/1 -> AEB_PedTrig/1
  Logical Operator3/1 -> Logical Operator7/2
  Logical Operator4/1 -> Compare To Constant13/1, Compare To Constant14/1
  Compare To Constant7/1 -> Logical Operator1/1
  Logical Operator/1 -> Logical Operator1/2
  AEBLonObjectType/1 -> Compare To Constant3/1, Compare To Constant12/1
  Compare To Constant1/1 -> Logical Operator/2
  Compare To Constant3/1 -> Logical Operator4/1
  Compare To Constant/1 -> Logical Operator/1
  Compare To Constant2/1 -> Logical Operator/3
  AEBfullFuncImpArb/1 -> Compare To Constant1/1
  EBAfullFuncImpArb/1 -> Compare To Constant/1
  Compare To Constant12/1 -> Logical Operator4/2
  AEBPartFuncImpArb/1 -> Compare To Constant2/1
  Compare To Constant8/1 -> Logical Operator3/1
  Logical Operator2/1 -> Logical Operator3/2
  Compare To Constant5/1 -> Logical Operator2/2
  cycAEBpartFuncImpArb/1 -> Compare To Constant6/1
  Compare To Constant6/1 -> Logical Operator2/3
  cycAEBfullFuncImpArb/1 -> Compare To Constant5/1
  Compare To Constant4/1 -> Logical Operator2/1
  pedAEBfullFuncImpArb/1 -> Compare To Constant4/1
  Logical Operator5/1 -> AEB_VehTrig/1
  Switch/1 -> AEB_JABrkTrig/1
  Constant3/1 -> Switch1/3
  Switch2/1 -> Switch/1
  Constant1/1 -> Switch1/1
  Switch1/1 -> Switch2/3
  Constant2/1 -> Switch2/1
  Compare To Constant10/1 -> Switch1/2
  Compare To Constant9/1 -> Switch2/2
  Constant/1 -> Switch/3
  Compare To Constant11/1 -> Switch/2
  JA_pedAEBfullFuncImpArb/1 -> Compare To Constant9/1
  AEBTrigger/1 -> Compare To Constant8/1, Compare To Constant11/1, Compare To Constant7/1  "AEBTrigger"
  JA_AEBfullFuncImpArb/1 -> Compare To Constant10/1

== system TosAeb/FcwAebJa_Step_sys/ALG_AEBSystem/ALG_AEBOutput/AEBS_IPHUTInfoCalc/AEB_TrigMap1 ==
  note: AEB_JABrkTrigMap
  note: AEB_PedTrigMap
  note: AEB_VehTrigMap
  note: 0x0:Not tirggered
      | 0x1:AEB_JA Function Triggered for vehicle
      | 0x2:AEB_JA Function Triggered for pedestrian
      | 0x3:reverse
  AEBTrigger : Inport
  AEBLonObjectType : Inport  Port=2
  EBAfullFuncImpArb : Inport  Port=3
  AEBfullFuncImpArb : Inport  Port=4
  AEBPartFuncImpArb : Inport  Port=5
  pedAEBfullFuncImpArb : Inport  Port=6
  cycAEBfullFuncImpArb : Inport  Port=7
  cycAEBpartFuncImpArb : Inport  Port=8
  JA_pedAEBfullFuncImpArb : Inport  Port=9
  JA_AEBfullFuncImpArb : Inport  Port=10
  Compare To Constant{,1..2,4..6,9..10} (8 alike) : simulink/Logic and Bit Operations/Compare To Constant  relop===  const=ModSts.ActiveState  OutDataTypeStr=boolean
  Compare To Constant{7..8,11,14} (4 alike) : simulink/Logic and Bit Operations/Compare To Constant  relop===  const=TRUE  OutDataTypeStr=boolean
  Compare To Constant12 : simulink/Logic and Bit Operations/Compare To Constant  relop===  const=ObjectTypeCopy.BICYCLE  OutDataTypeStr=boolean
  Compare To Constant13 : simulink/Logic and Bit Operations/Compare To Constant  relop===  const=FALSE  OutDataTypeStr=boolean
  Compare To Constant3 : simulink/Logic and Bit Operations/Compare To Constant  relop===  const=ObjectTypeCopy.PEDESTRIAN  OutDataTypeStr=boolean
  Constant1 : Constant  OutDataTypeStr=uint8
  Constant3 : Constant  Value=0  OutDataTypeStr=uint8
  Logical Operator : Logic  Operator=OR  Inputs=3  AllPortsSameDT=off  OutDataTypeStr=boolean
  Logical Operator{1,3,5..6,8} (5 alike) : Logic  AllPortsSameDT=off  OutDataTypeStr=boolean
  Logical Operator2 : Logic  Operator=OR  Inputs=4  AllPortsSameDT=off  OutDataTypeStr=boolean
  Logical Operator4 : Logic  Operator=OR  AllPortsSameDT=off  OutDataTypeStr=boolean
  Logical Operator7 : Logic  Operator=OR  AllPortsSameDT=off  OutDataTypeStr=boolean
  Switch1 : Switch  Criteria=u2 ~= 0
  AEB_VehTrig : Outport
  AEB_PedTrig : Outport  Port=2
  AEB_JABrkTrig : Outport  Port=3
  wires:
  JA_AEBfullFuncImpArb/1 -> Compare To Constant10/1
  AEBTrigger/1 -> Compare To Constant7/1, Compare To Constant11/1, Compare To Constant8/1  "AEBTrigger"
  JA_pedAEBfullFuncImpArb/1 -> Compare To Constant9/1
  Compare To Constant11/1 -> Logical Operator8/1
  Compare To Constant9/1 -> Logical Operator2/4
  Compare To Constant10/1 -> Switch1/2
  Switch1/1 -> Logical Operator8/2
  Constant1/1 -> Switch1/1
  Constant3/1 -> Switch1/3
  Logical Operator8/1 -> AEB_JABrkTrig/1
  Logical Operator5/1 -> AEB_VehTrig/1
  pedAEBfullFuncImpArb/1 -> Compare To Constant4/1
  Compare To Constant4/1 -> Logical Operator2/1
  cycAEBfullFuncImpArb/1 -> Compare To Constant5/1
  Compare To Constant6/1 -> Logical Operator2/3
  cycAEBpartFuncImpArb/1 -> Compare To Constant6/1
  Compare To Constant5/1 -> Logical Operator2/2
  Logical Operator2/1 -> Logical Operator3/2
  Compare To Constant8/1 -> Logical Operator3/1
  AEBPartFuncImpArb/1 -> Compare To Constant2/1
  Compare To Constant12/1 -> Logical Operator4/2
  EBAfullFuncImpArb/1 -> Compare To Constant/1
  AEBfullFuncImpArb/1 -> Compare To Constant1/1
  Compare To Constant2/1 -> Logical Operator/3
  Compare To Constant/1 -> Logical Operator/1
  Compare To Constant3/1 -> Logical Operator4/1
  Compare To Constant1/1 -> Logical Operator/2
  AEBLonObjectType/1 -> Compare To Constant12/1, Compare To Constant3/1
  Logical Operator/1 -> Logical Operator1/2
  Compare To Constant7/1 -> Logical Operator1/1
  Logical Operator4/1 -> Compare To Constant14/1, Compare To Constant13/1
  Logical Operator3/1 -> Logical Operator7/2
  Logical Operator7/1 -> AEB_PedTrig/1
  Logical Operator6/1 -> Logical Operator7/1
  Compare To Constant14/1 -> Logical Operator6/1
  Logical Operator1/1 -> [AEB_VehTrig], Logical Operator5/1
  Compare To Constant13/1 -> Logical Operator5/2
  [AEB_VehTrig] -> Logical Operator6/2

== system TosAeb/FcwAebJa_Step_sys/ALG_AEBSystem/ALG_AEBOutput/AEBS_IPHUTInfoCalc/AR_HUD_InfoMap ==
  note: AEB_LePedDetn
      |
      | AEB_LePedTyp
      |
      | AEB_LePedHeading
      |
      | AEB_LePedDx
      |
      | AEB_LePedDy
      |
      | AEB_LePed_collisionprob
      |
      | AEB_RiPedDetn
      |
      | AEB_RiPedTyp
      |
      | AEB_RiPedHeading
      |
      | AEB_RiPedDx
      |
      | AEB_RiPedDy
      |
      | AEB_RiPed_collisionprob
      |
      | AEB_LeCyclistDetn
      |
      | AEB_LeCyclistTyp
      |
      | AEB_LeCyclistHeading
      |
      | AEB_LeCyclistDx
      |
      | AEB_LeCyclistDy
      |
      | AEB_LeCyclist_collisionprob
      |
      | AEB_RiCyclistDetn
      |
      | AEB_RiCyclistTyp
      |
      | AEB_RiCyclistHeading
      |
      | AEB_RiCyclistDx
      |
      | AEB_RiCyclistDy
      |
      | AEB_RiCyclist_collisionprob
      |
      | FCWAEB_longTTC
      |
      | FCWAEB_crossingTTC
      |
      | FCWAEB_JATTC
  FCWAEB_longTTC : Inport
  VehicleConfigAEBPed : Inport  Port=2
  VehicleConfigAEBCyc : Inport  Port=3
  pedPreWarningFuncImpArb : Inport  Port=4
  pedPrefillFuncImpArb : Inport  Port=5
  pedABAFuncImpArb : Inport  Port=6
  pedAEBfullFuncImpArb : Inport  Port=7
  cycPrefillFuncImpArb : Inport  Port=8
  cycAEBpartFuncImpArb : Inport  Port=9
  cycAEBfullFuncImpArb : Inport  Port=10
  latPedCalcInfo : Inport  Port=11
  latCycCalcInfo : Inport  Port=12
  AEB_Cyc_collisionprob : Subsystem
  AEB_LeCycFlow : Stateflow chart  TreatAsAtomicUnit=on
  AEB_LePedFlow : Stateflow chart  TreatAsAtomicUnit=on
  AEB_Ped_collisionprob : Subsystem
  AEB_RiCycFlow : Stateflow chart  TreatAsAtomicUnit=on
  AEB_RiPedFlow : Stateflow chart  TreatAsAtomicUnit=on
  Bus Creator : BusCreator  Inputs=27
  Constant : Constant  Value=0
  Constant1 : Constant  Value=0
  AR_HUD_Info : Outport
  wires:
  VehicleConfigAEBCyc/1 -> AEB_RiCycFlow/2(VehicleConfigAEBCyc), AEB_LeCycFlow/2(VehicleConfigAEBCyc)
  VehicleConfigAEBPed/1 -> AEB_RiPedFlow/2(VehicleConfigAEBPed), AEB_LePedFlow/2(VehicleConfigAEBPed)
  AEB_Ped_collisionprob/1(AEB_Ped_collisionprob) -> AEB_RiPedFlow/1(AEB_Ped_collisionprob), AEB_LePedFlow/3(AEB_Ped_collisionprob)
  AEB_Cyc_collisionprob/1(AEB_Cyc_collisionprob) -> AEB_LeCycFlow/3(AEB_Cyc_collisionprob), AEB_RiCycFlow/1(AEB_Cyc_collisionprob)
  pedAEBfullFuncImpArb/1 -> AEB_Ped_collisionprob/4(pedAEBfullFuncImpArb)
  pedABAFuncImpArb/1 -> AEB_Ped_collisionprob/3(pedABAFuncImpArb)
  pedPrefillFuncImpArb/1 -> AEB_Ped_collisionprob/2(pedPrefillFuncImpArb)
  pedPreWarningFuncImpArb/1 -> AEB_Ped_collisionprob/1(pedPreWarningFuncImpArb)
  cycAEBfullFuncImpArb/1 -> AEB_Cyc_collisionprob/3(cycAEBfullFuncImpArb)
  cycAEBpartFuncImpArb/1 -> AEB_Cyc_collisionprob/2(cycAEBpartFuncImpArb)
  cycPrefillFuncImpArb/1 -> AEB_Cyc_collisionprob/1(cycPrefillFuncImpArb)
  Constant1/1 -> Bus Creator/27  "FCWAEB_JATTC"
  Constant/1 -> Bus Creator/26  "FCWAEB_crossingTTC"
  FCWAEB_longTTC/1 -> Bus Creator/25  "FCWAEB_longTTC"
  AEB_RiCycFlow/4(AEB_RiCycDx) -> Bus Creator/22  "AEB_RiCyclistDx"
  AEB_RiCycFlow/3(AEB_RiCycHeading) -> Bus Creator/21  "AEB_RiCyclistHeading"
  AEB_RiCycFlow/6(AEB_RiCyc_collisionprob) -> Bus Creator/24  "AEB_RiCyclist_collisionprob"
  AEB_RiCycFlow/5(AEB_RiCycDy) -> Bus Creator/23  "AEB_RiCyclistDy"
  AEB_RiCycFlow/2(AEB_RiCycTyp) -> Bus Creator/20  "AEB_RiCyclistTyp"
  AEB_RiCycFlow/1(AEB_RiCycDetn) -> Bus Creator/19  "AEB_RiCyclistDetn"
  AEB_LeCycFlow/6(AEB_LeCyc_collisionprob) -> Bus Creator/18  "AEB_LeCyclist_collisionprob"
  AEB_LeCycFlow/5(AEB_LeCycDy) -> Bus Creator/17  "AEB_LeCyclistDy"
  AEB_LeCycFlow/4(AEB_LeCycDx) -> Bus Creator/16  "AEB_LeCyclistDx"
  AEB_LeCycFlow/3(AEB_LeCycHeading) -> Bus Creator/15  "AEB_LeCyclistHeading"
  AEB_LeCycFlow/2(AEB_LeCycTyp) -> Bus Creator/14  "AEB_LeCyclistTyp"
  AEB_LeCycFlow/1(AEB_LeCycDetn) -> Bus Creator/13  "AEB_LeCyclistDetn"
  AEB_RiPedFlow/6(AEB_RiPed_collisionprob) -> Bus Creator/12  "AEB_RiPed_collisionprob"
  AEB_RiPedFlow/5(AEB_RiPedDy) -> Bus Creator/11  "AEB_RiPedDy"
  AEB_RiPedFlow/4(AEB_RiPedDx) -> Bus Creator/10  "AEB_RiPedDx"
  AEB_RiPedFlow/3(AEB_RiPedHeading) -> Bus Creator/9  "AEB_RiPedHeading"
  AEB_RiPedFlow/2(AEB_RiPedTyp) -> Bus Creator/8  "AEB_RiPedTyp"
  AEB_LePedFlow/5(AEB_LePedDy) -> Bus Creator/5  "AEB_LePedDy"
  AEB_LePedFlow/4(AEB_LePedDx) -> Bus Creator/4  "AEB_LePedDx"
  AEB_LePedFlow/3(AEB_LePedHeading) -> Bus Creator/3  "AEB_LePedHeading"
  AEB_LePedFlow/2(AEB_LePedTyp) -> Bus Creator/2  "AEB_LePedTyp"
  AEB_LePedFlow/1(AEB_LePedDetn) -> Bus Creator/1  "AEB_LePedDetn"
  Bus Creator/1 -> AR_HUD_Info/1
  AEB_RiPedFlow/1(AEB_RiPedDetn) -> Bus Creator/7  "AEB_RiPedDetn"
  AEB_LePedFlow/6(AEB_LePed_collisionprob) -> Bus Creator/6  "AEB_LePed_collisionprob"
  latCycCalcInfo/1 -> AEB_LeCycFlow/1(latCycCalcInfo), AEB_RiCycFlow/3(latCycCalcInfo)
  latPedCalcInfo/1 -> AEB_RiPedFlow/3(latPedCalcInfo), AEB_LePedFlow/1(latPedCalcInfo)

== system TosAeb/FcwAebJa_Step_sys/ALG_AEBSystem/ALG_AEBOutput/AEBS_IPHUTInfoCalc/FCW_AEB_FctStsCalc ==
  AEB_VehResp : Inport
  AEB_PedResp : Inport  Port=2
  AEB_JAResp : Inport  Port=3
  FCW_AEB_ErrorState : Inport  Port=4
  AEB_PEDP_ErrorState : Inport  Port=5
  AEB_JA_ErrorState : Inport  Port=6
  EspFuncOffSts : Inport  Port=7
  TrailerSts : Inport  Port=8
  FCW_AEB_FctStsCalc : Stateflow chart  TreatAsAtomicUnit=on
  FCW_AEB_VehFctSts : Outport
  FCW_AEB_PedFctSts : Outport  Port=2
  AEB_JAFuncSts : Outport  Port=3
  wires:
  AEB_VehResp/1 -> FCW_AEB_FctStsCalc/1(AEB_VehResp)
  AEB_JAResp/1 -> FCW_AEB_FctStsCalc/3(AEB_JAResp)
  AEB_PedResp/1 -> FCW_AEB_FctStsCalc/2(AEB_PedResp)
  TrailerSts/1 -> FCW_AEB_FctStsCalc/8(TrailerSts)
  FCW_AEB_ErrorState/1 -> FCW_AEB_FctStsCalc/4(FCW_AEB_ErrorState)
  EspFuncOffSts/1 -> FCW_AEB_FctStsCalc/7(EspFuncOffSts)
  AEB_JA_ErrorState/1 -> FCW_AEB_FctStsCalc/6(AEB_JA_ErrorState)
  AEB_PEDP_ErrorState/1 -> FCW_AEB_FctStsCalc/5(AEB_PEDP_ErrorState)
  FCW_AEB_FctStsCalc/2(FCW_AEB_PedFctSts) -> FCW_AEB_PedFctSts/1
  FCW_AEB_FctStsCalc/3(AEB_JAFuncSts) -> AEB_JAFuncSts/1
  FCW_AEB_FctStsCalc/1(FCW_AEB_VehFctSts) -> FCW_AEB_VehFctSts/1

== system TosAeb/FcwAebJa_Step_sys/ALG_AEBSystem/ALG_AEBOutput/AEBS_IPHUTInfoCalc/FCW_WarnMap ==
  note: 0x0:No warning
      | 0x1:Warning
  note: 0x0:No warning
      | 0x1:Latent warning
  preWarningTrigger : Inport
  latentWarningTrigger : Inport  Port=2
  AEBTrigger : Inport  Port=3
  Compare To Constant : simulink/Logic and Bit Operations/Compare To Constant  relop===  const=TRUE  OutDataTypeStr=boolean
  Compare To Constant1 : simulink/Logic and Bit Operations/Compare To Constant  relop===  const=TRUE  OutDataTypeStr=boolean
  Compare To Constant2 : simulink/Logic and Bit Operations/Compare To Constant  relop===  const=uint8(0)  OutDataTypeStr=boolean
  Constant : Constant  OutDataTypeStr=uint8
  Constant1 : Constant  OutDataTypeStr=uint8
  Constant2 : Constant  Value=0  OutDataTypeStr=uint8
  Constant3 : Constant  Value=P_AEB_AWB_ConfigSet
  Constant4 : Constant  Value=0  OutDataTypeStr=uint8
  Logical Operator : Logic  Operator=OR  AllPortsSameDT=off  OutDataTypeStr=boolean
  Logical Operator1 : Logic  AllPortsSameDT=off  OutDataTypeStr=boolean
  Switch : Switch  Criteria=u2 ~= 0
  Switch1 : Switch  Criteria=u2 ~= 0
  FCW_Warn : Outport
  Latten_Warn : Outport  Port=2
  wires:
  Logical Operator1/1 -> Logical Operator/2
  AEBTrigger/1 -> Logical Operator1/2
  Compare To Constant2/1 -> Logical Operator1/1
  Constant3/1 -> Compare To Constant2/1
  Logical Operator/1 -> Compare To Constant/1
  Switch/1 -> FCW_Warn/1
  Constant2/1 -> Switch1/3
  Constant1/1 -> Switch1/1
  Compare To Constant1/1 -> Switch1/2
  latentWarningTrigger/1 -> Compare To Constant1/1
  Constant/1 -> Switch/1
  Compare To Constant/1 -> Switch/2
  preWarningTrigger/1 -> Logical Operator/1
  Constant4/1 -> Switch/3
  Switch1/1 -> Latten_Warn/1

== system TosAeb/FcwAebJa_Step_sys/ALG_AEBSystem/ALG_AEBOutput/AEB_EDR_JAPED_INFOMap/If Action Subsystem ==
  Action Port : ActionPort  ActionPortLabel=if(u1)
  Bus Creator : BusCreator  Inputs=25  OutDataTypeStr=Bus: AEB_EDR_LATPED_INFO_BUS
      settings: InheritFromInputs=off
  Constant{,1..16,20..27} (25 alike) : Constant  Value=0
  Data Type Conversion{,5..24} (21 alike) : DataTypeConversion
  Data Type Conversion{1..4} (4 alike) : DataTypeConversion  OutDataTypeStr=uint8
  AEB_EDR_JAPED_INFO : Outport  OutDataTypeStr=Bus: AEB_EDR_JAPED_INFO_BUS
  wires:
  Constant14/1 -> Data Type Conversion14/1
  Constant15/1 -> Data Type Conversion15/1
  Constant21/1 -> Data Type Conversion17/1
  Constant20/1 -> Data Type Conversion16/1
  Constant23/1 -> Data Type Conversion19/1
  Constant24/1 -> Data Type Conversion20/1
  Constant22/1 -> Data Type Conversion18/1
  Constant27/1 -> Data Type Conversion23/1
  Constant16/1 -> Data Type Conversion24/1
  Constant26/1 -> Data Type Conversion22/1
  Constant25/1 -> Data Type Conversion21/1
  Constant7/1 -> Data Type Conversion9/1
  Constant8/1 -> Data Type Conversion4/1
  Constant10/1 -> Data Type Conversion10/1
  Constant9/1 -> Data Type Conversion1/1
  Constant12/1 -> Data Type Conversion12/1
  Constant13/1 -> Data Type Conversion13/1
  Constant11/1 -> Data Type Conversion11/1
  Constant4/1 -> Data Type Conversion6/1
  Constant6/1 -> Data Type Conversion8/1
  Constant5/1 -> Data Type Conversion7/1
  Constant2/1 -> Data Type Conversion3/1
  Constant3/1 -> Data Type Conversion5/1
  Constant1/1 -> Data Type Conversion/1
  Constant/1 -> Data Type Conversion2/1
  Bus Creator/1 -> AEB_EDR_JAPED_INFO/1
  Data Type Conversion2/1 -> Bus Creator/1  "FusionType"
  Data Type Conversion20/1 -> Bus Creator/21  "dyVariance"
  Data Type Conversion3/1 -> Bus Creator/3  "ObjectType"
  Data Type Conversion1/1 -> Bus Creator/10  "ObjectMovingOrientation"
  Data Type Conversion4/1 -> Bus Creator/9  "ObjectMovingState"
  Data Type Conversion17/1 -> Bus Creator/18  "ax"
  Data Type Conversion8/1 -> Bus Creator/7  "ObjectHight"
  Data Type Conversion9/1 -> Bus Creator/8  "ObjectYawAngle"
  Data Type Conversion7/1 -> Bus Creator/6  "ObjectWidth"
  Data Type Conversion/1 -> Bus Creator/2  "ObjectID"
  Data Type Conversion16/1 -> Bus Creator/17  "vy"
  Data Type Conversion6/1 -> Bus Creator/5  "ObjectLength"
  Data Type Conversion22/1 -> Bus Creator/23  "ObjectIsObstacleProb"
  Data Type Conversion12/1 -> Bus Creator/13  "dx"
  Data Type Conversion21/1 -> Bus Creator/22  "ObjectExistProb"
  Data Type Conversion18/1 -> Bus Creator/19  "ay"
  Data Type Conversion10/1 -> Bus Creator/11  "dxFisrtDetection"
  Data Type Conversion23/1 -> Bus Creator/24  "ObjectMeasurementStatus"
  Data Type Conversion24/1 -> Bus Creator/25  "ObjectLifeCycle"
  Data Type Conversion19/1 -> Bus Creator/20  "dxVariance"
  Data Type Conversion14/1 -> Bus Creator/15  "dz"
  Data Type Conversion11/1 -> Bus Creator/12  "dyFisrtDetection"
  Data Type Conversion15/1 -> Bus Creator/16  "vx"
  Data Type Conversion5/1 -> Bus Creator/4  "ObjectTypeCameraConfirmed"
  Data Type Conversion13/1 -> Bus Creator/14  "dy"
